simulink model "non_linear_final"  (R2021b) (continued)
== system non_linear_final/Directional Control Valve == (continued)
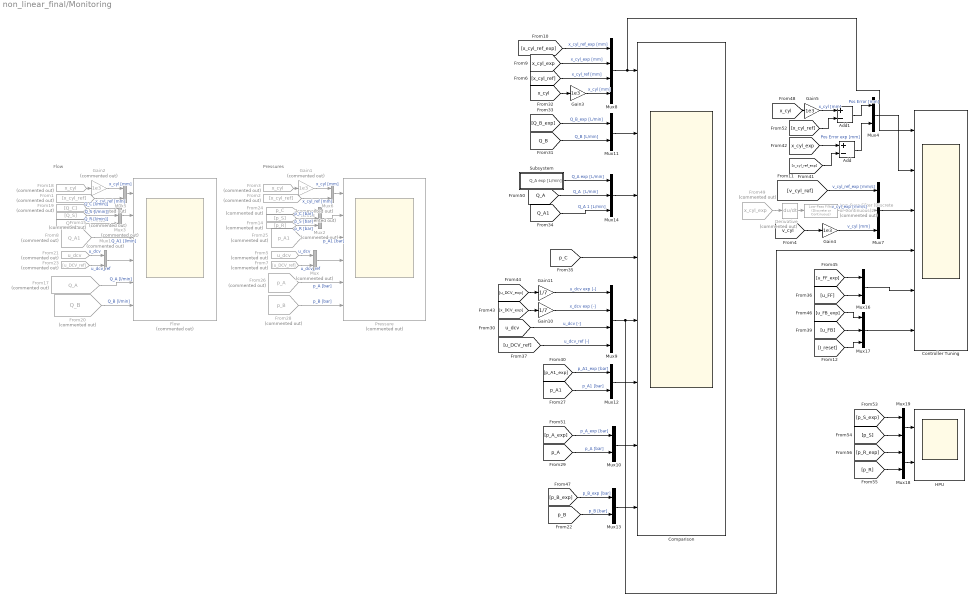
== system non_linear_final/Monitoring ==
  note: Pressures
  note: Flow
  Add : Sum  Inputs=+-
  Add1 : Sum  Inputs=+-
  Comparison : Scope  NumInputPorts=8
  Controller Tuning : Scope  NumInputPorts=6
  Derivative : Derivative  Commented=on
  Flow : Scope  Commented=on  NumInputPorts=6
  From1 : From  Commented=on  GotoTag=x_cyl_ref  TagVisibility=global
  From13 : From  Commented=on  GotoTag=p_S  TagVisibility=global
  From14 : From  Commented=on  GotoTag=p_R  TagVisibility=global
  From15 : From  Commented=on  GotoTag=Q_S  TagVisibility=global
  From16 : From  Commented=on  GotoTag=Q_R  TagVisibility=global
  From17 : From  Commented=on  GotoTag=Q_A  TagVisibility=global
  From18 : From  Commented=on  GotoTag=x_cyl  TagVisibility=global
  From19 : From  Commented=on  GotoTag=Q_C  TagVisibility=global
  From2 : From  Commented=on  GotoTag=x_cyl_ref  TagVisibility=global
  From20 : From  Commented=on  GotoTag=Q_B  TagVisibility=global
  From21 : From  Commented=on  GotoTag=u_dcv  TagVisibility=global
  From23 : From  Commented=on  GotoTag=u_DCV_ref  TagVisibility=global
  From24 : From  Commented=on  GotoTag=p_C  TagVisibility=global
  From25 : From  Commented=on  GotoTag=p_A1  TagVisibility=global
  From26 : From  Commented=on  GotoTag=p_A  TagVisibility=global
  From28 : From  Commented=on  GotoTag=p_B  TagVisibility=global
  From3 : From  Commented=on  GotoTag=x_cyl  TagVisibility=global
  From49 : From  Commented=on  GotoTag=x_cyl_exp  TagVisibility=global
  From5 : From  Commented=on  GotoTag=u_dcv  TagVisibility=global
  From7 : From  Commented=on  GotoTag=u_DCV_ref  TagVisibility=global
  From8 : From  Commented=on  GotoTag=Q_A1  TagVisibility=global
  Gain1 : Gain  Commented=on  Gain=1e3
  Gain10 : Gain  Gain=1/7
  Gain11 : Gain  Gain=1/7
  Gain2 : Gain  Commented=on  Gain=1e3
  Gain3 : Gain  Gain=1e3
  Gain4 : Gain  Gain=1e3
  Gain5 : Gain  Gain=1e3
  HPU : Scope  NumInputPorts=2
  Low-Pass Filter (Discrete or Continuous)2 : eeGeneralControl/Low-Pass Filter (Discrete or Continuous)  Commented=on  K=1  T=0.1  B=150  A=-150  init_option=Inherited from block input  x0=0  Ts=0
  Mux{,1,5..6} (4 alike) : Mux  Commented=on  Inputs=2
  Mux{4,10..13,16,18..19} (8 alike) : Mux  Inputs=2
  Mux14 : Mux  Inputs=3
  Mux17 : Mux  Inputs=3
  Mux2 : Mux  Commented=on  Inputs=3
  Mux3 : Mux  Commented=on  Inputs=3
  Mux7 : Mux  Inputs=3
  Mux8 : Mux
  Mux9 : Mux
  Pressure : Scope  Commented=on  NumInputPorts=6
  Subsystem : Subsystem
  wires:
  From28/1 -> Pressure/6  "p_B [bar]"
  From8/1 -> Flow/3  "Q_A1 [l/min]"
  From21/1 -> Mux1/1  "u_dcv"
  From17/1 -> Flow/5  "Q_A [l/min]"
  From26/1 -> Pressure/5  "p_A [bar]"
  From23/1 -> Mux1/2  "u_dcv_ref"
  From20/1 -> Flow/6  "Q_B [l/min]"
  Mux/1 -> Pressure/4
  From2/1 -> Mux6/2  "x_cyl_ref [mm]"
  From16/1 -> Mux3/3  "Q_R [l/min]]"
  From3/1 -> Gain1/1
  From24/1 -> Mux2/1  "p_C [bar]"
  From7/1 -> Mux/2  "u_dcv_ref"
  Mux1/1 -> Flow/4
  From25/1 -> Pressure/3  "p_A1 [bar]"
  Mux3/1 -> Flow/2
  Mux2/1 -> Pressure/2
  From5/1 -> Mux/1  "u_dcv"
  Mux5/1 -> Flow/1
  From14/1 -> Mux2/3  "p_R [bar]"
  From1/1 -> Mux5/2  "x_cyl_ref [mm]"
  From15/1 -> Mux3/2  "Q_S [l/min]]"
  From18/1 -> Gain2/1
  Gain2/1 -> Mux5/1  "x_cyl [mm]"
  From19/1 -> Mux3/1  "Q_C [l/min]]"
  From13/1 -> Mux2/2  "p_S [bar]"
  Gain1/1 -> Mux6/1  "x_cyl [mm]"
  Mux6/1 -> Pressure/1
  Mux8/1 -> Controller Tuning/1, Comparison/1
  [x_cyl_ref] -> Mux8/3  "x_cyl_ref [mm]"
  [x_cyl] -> Gain3/1
  Gain3/1 -> Mux8/4  "x_cyl [mm]"
  [x_cyl_ref_exp] -> Mux8/1  "x_cyl_ref_exp [mm]"
  [x_cyl_exp] -> Mux8/2  "x_cyl_exp [mm]"
  [Q_B] -> Mux11/2  "Q_B [L/min]"
  [Q_B_exp] -> Mux11/1  "Q_B_exp [L/min]"
  Mux11/1 -> Comparison/2
  [p_R] -> Mux18/2
  [p_R_exp] -> Mux18/1
  Mux18/1 -> HPU/2
  [p_S_exp] -> Mux19/1
  [p_S] -> Mux19/2
  Mux19/1 -> HPU/1
  [p_C] -> Comparison/4
  Mux17/1 -> Controller Tuning/6
  [u_FB] -> Mux17/2
  [u_FF] -> Mux16/2
  [u_FF_exp] -> Mux16/1
  [u_FB_exp] -> Mux17/1
  Mux16/1 -> Controller Tuning/5
  [x_cyl_exp] -> Add/1
  [x_cyl_ref] -> Add1/2
  [x_cyl_ref_exp] -> Add/2
  Gain5/1 -> Add1/1  "x_cyl [mm]"
  [x_cyl] -> Gain5/1
  Add/1 -> Mux4/2  "Pos Error exp [mm]"
  Add1/1 -> Mux4/1  "Pos Error [mm]"
  Mux4/1 -> Controller Tuning/2
  [I_reset] -> Mux17/3
  [x_DCV_exp] -> Gain10/1
  Gain10/1 -> Mux9/2  "x_dcv exp [-]"
  [u_DCV_ref] -> Mux9/4  "u_dcv_ref [-]"
  [u_dcv] -> Mux9/3  "u_dcv [-]"
  Gain11/1 -> Mux9/1  "u_dcv exp [-]"
  [p_A1_exp] -> Mux12/1  "p_A1_exp [bar]"
  [p_A1] -> Mux12/2  "p_A1 [bar]"
  Mux12/1 -> Comparison/6
  Mux9/1 -> Controller Tuning/4, Comparison/5
  Low-Pass Filter (Discrete or Continuous)2/1 -> Mux7/2  "v_cyl_exp [mm/s]"
  Mux7/1 -> Controller Tuning/3
  Derivative/1 -> Low-Pass Filter (Discrete or Continuous)2/1
  [v_cyl_ref] -> Mux7/1  "v_cyl_ref_exp [mm/s]"
  [v_cyl] -> Gain4/1
  From49/1 -> Derivative/1
  Gain4/1 -> Mux7/3  "v_cyl [mm]"
  [Q_A1] -> Mux14/3  "Q_A 1 [L/min]"
  Subsystem/1(Q_A exp [L/min]) -> Mux14/1  "Q_A exp [L/min]"
  [Q_A] -> Mux14/2  "Q_A  [L/min]"
  Mux14/1 -> Comparison/3
  [u_DCV_exp] -> Gain11/1
  [p_B] -> Mux13/2  "p_B [bar]"
  [p_B_exp] -> Mux13/1  "p_B_exp [bar]"
  [p_A] -> Mux10/2  "p_A [bar]"
  [p_A_exp] -> Mux10/1  "p_A_exp [bar]"
  Mux13/1 -> Comparison/8
  Mux10/1 -> Comparison/7

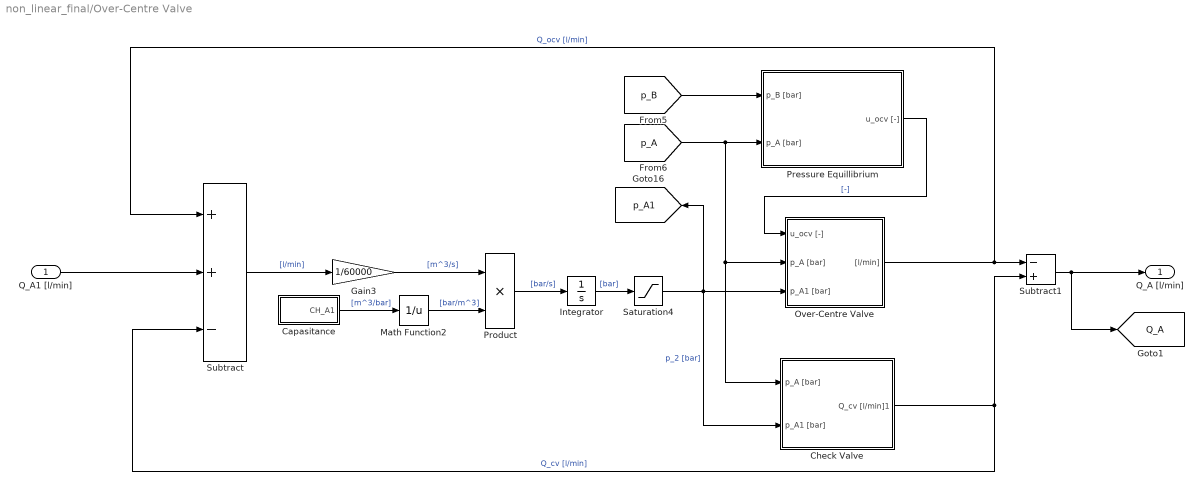
== system non_linear_final/Over-Centre Valve ==
  Q_A1 [l/min] : Inport
  Capasitance : Subsystem
  Check Valve : Subsystem
  Gain3 : Gain  Gain=1/60000
  Integrator : Integrator  InitialCondition=p0_A1
  Math Function2 : Math  Operator=reciprocal  SignedPower=on  SaturateOnIntegerOverflow=on
  Over-Centre Valve : Subsystem
  Pressure Equillibrium : Subsystem
  Product : Product
  Saturation4 : Saturate  UpperLimit=200  LowerLimit=p_R  ZeroCross=off
  Subtract : Sum  Inputs=++-
  Subtract1 : Sum  Inputs=-+
  Q_A [l/min] : Outport  PortDimensions=1  VectorParamsAs1DForOutWhenUnconnected=off
  tag scope: Q_A=global, p_A1=global
  wires:
  [p_B] -> Pressure Equillibrium/1(p_B [bar])
  Pressure Equillibrium/1(u_ocv [-]) -> Over-Centre Valve/1(u_ocv [-])  "[-]"
  Check Valve/1(Q_cv [l/min]1) -> Subtract/3, Subtract1/2  "Q_cv [l/min]"
  Over-Centre Valve/1("[l/min]") -> Subtract1/1, Subtract/1  "Q_ocv [l/min]"
  Saturation4/1 -> Check Valve/2(p_A1 [bar]), Over-Centre Valve/3(p_A1 [bar]), [p_A1]  "p_2 [bar]"
  Subtract/1 -> Gain3/1  "[l/min]"
  Q_A1 [l/min]/1 -> Subtract/2
  Product/1 -> Integrator/1  "[bar/s]"
  Integrator/1 -> Saturation4/1  "[bar]"
  Gain3/1 -> Product/1  "[m^3/s]"
  Subtract1/1 -> [Q_A], Q_A [l/min]/1
  [p_A] -> Pressure Equillibrium/2(p_A [bar]), Over-Centre Valve/2(p_A [bar]), Check Valve/1(p_A [bar])
  Capasitance/1("CH_A1 ") -> Math Function2/1  "[m^3/bar]"
  Math Function2/1 -> Product/2  "[bar/m^3]"

== system non_linear_final/Pressure Compensator1 ==
  p_S [bar] : Inport
  Compensator From Morten Bak Paper : MATLAB Function  TreatAsAtomicUnit=on
  "Compensator setting [bar] " : Constant  Value=p_pc_set
  Transfer Fcn1 : TransferFcn  Denominator=[tau_pc 1]
  p_C [bar] : Outport  VectorParamsAs1DForOutWhenUnconnected=off
  tag scope: p_C=global
  wires:
  [p_LS] -> Compensator From Morten Bak Paper/3(p_LS)
  "Compensator setting [bar] "/1 -> Compensator From Morten Bak Paper/1(p0)
  p_S [bar]/1 -> Compensator From Morten Bak Paper/2(p_S)
  Compensator From Morten Bak Paper/1(p_C) -> Transfer Fcn1/1  "p_C [bar]"
  Transfer Fcn1/1 -> [p_C], p_C [bar]/1  "u_ocv_ref [-]"

== system non_linear_final/Rigid Mechanical System - Green Crane Arm ==
  note: Green Crane (Lagrangian mechanics)
  Force_Input : Inport
  Divide : Product  Inputs=*/
  Lasse Schmidt : MATLAB Function  TreatAsAtomicUnit=on
  Math Function : Math  Operator=square  SignedPower=on  SaturateOnIntegerOverflow=on
  Position : Integrator  InitialCondition=x_cyl_0
  Product : Product
  Scope : Scope
  Sum2 : Sum  Inputs=+--
  Velocity : Integrator  InitialCondition=v_cyl_0
  tag scope: v_cyl=global, x_cyl=global
  wires:
  Velocity/1 -> [v_cyl], Position/1, Math Function/1  "[m/s]"
  Lasse Schmidt/3(Meq) -> Divide/2  "[kg]"
  Sum2/1 -> Divide/1  "[N]"
  Force_Input/1 -> Sum2/1
  Divide/1 -> Velocity/1  "[m/s^2]"
  Lasse Schmidt/1(Geq) -> Sum2/2  "[N]"
  Position/1 -> [x_cyl], Lasse Schmidt/1(x)  "[m]"
  Lasse Schmidt/2(Ceq) -> Product/1
  Math Function/1 -> Product/2
  Product/1 -> Sum2/3, Scope/1  "[N]"

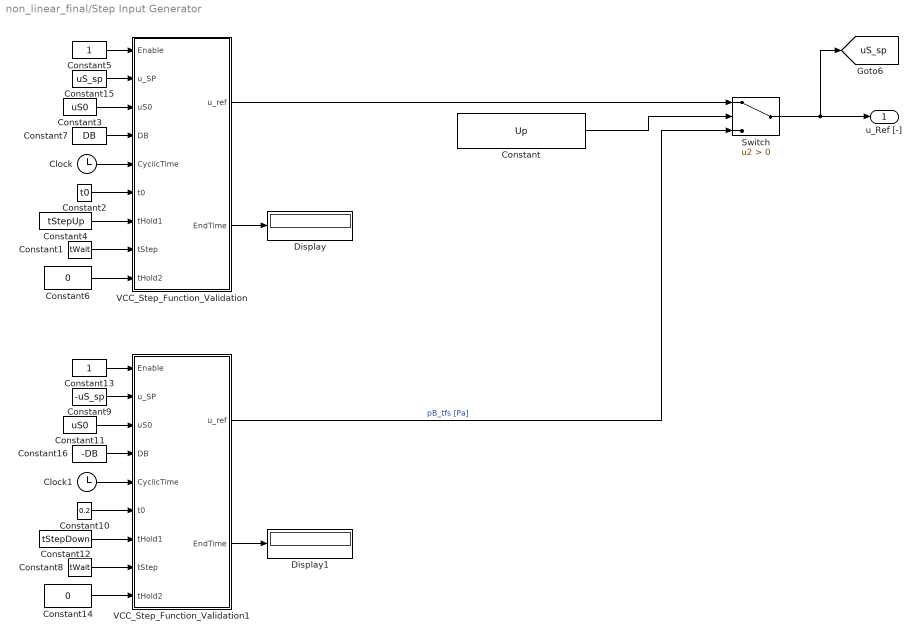
== system non_linear_final/Step Input Generator ==
  Clock : Clock
  Clock1 : Clock
  Constant : Constant  Value=Up
  Constant1 : Constant  Value=tWait
  Constant10 : Constant  Value=0.2
  Constant11 : Constant  Value=uS0
  Constant12 : Constant  Value=tStepDown
  Constant13 : Constant  OutDataTypeStr=boolean
  Constant14 : Constant  Value=0
  Constant15 : Constant  Value=uS_sp
  Constant16 : Constant  Value=-DB
  Constant2 : Constant  Value=t0
  Constant3 : Constant  Value=uS0
  Constant4 : Constant  Value=tStepUp
  Constant5 : Constant  OutDataTypeStr=boolean
  Constant6 : Constant  Value=0
  Constant7 : Constant  Value=DB
  Constant8 : Constant  Value=tWait
  Constant9 : Constant  Value=-uS_sp
  Display : Display
  Display1 : Display
  Switch : Switch  Criteria=u2 > Threshold
  VCC_Step_Function_Validation : Subsystem  TreatAsAtomicUnit=on
  VCC_Step_Function_Validation1 : Subsystem  TreatAsAtomicUnit=on
  u_Ref [-] : Outport  VectorParamsAs1DForOutWhenUnconnected=off
  tag scope: uS_sp=global
  wires:
  VCC_Step_Function_Validation/2(EndTime) -> Display/1
  Constant4/1 -> VCC_Step_Function_Validation/7(tHold1)
  Constant1/1 -> VCC_Step_Function_Validation/8(tStep)
  Clock/1 -> VCC_Step_Function_Validation/5(CyclicTime)
  Constant2/1 -> VCC_Step_Function_Validation/6(t0)
  Constant15/1 -> VCC_Step_Function_Validation/2(u_SP)
  VCC_Step_Function_Validation/1(u_ref) -> Switch/1
  Constant3/1 -> VCC_Step_Function_Validation/3(uS0)
  Constant6/1 -> VCC_Step_Function_Validation/9(tHold2)
  Constant5/1 -> VCC_Step_Function_Validation/1(Enable)
  Constant7/1 -> VCC_Step_Function_Validation/4(DB)
  Switch/1 -> [uS_sp], u_Ref [-]/1
  Constant/1 -> Switch/2
  VCC_Step_Function_Validation1/1(u_ref) -> Switch/3  "pB_tfs [Pa]"
  Constant16/1 -> VCC_Step_Function_Validation1/4(DB)
  Constant13/1 -> VCC_Step_Function_Validation1/1(Enable)
  Constant11/1 -> VCC_Step_Function_Validation1/3(uS0)
  Constant12/1 -> VCC_Step_Function_Validation1/7(tHold1)
  Clock1/1 -> VCC_Step_Function_Validation1/5(CyclicTime)
  VCC_Step_Function_Validation1/2(EndTime) -> Display1/1
  Constant9/1 -> VCC_Step_Function_Validation1/2(u_SP)
  Constant14/1 -> VCC_Step_Function_Validation1/9(tHold2)
  Constant10/1 -> VCC_Step_Function_Validation1/6(t0)
  Constant8/1 -> VCC_Step_Function_Validation1/8(tStep)

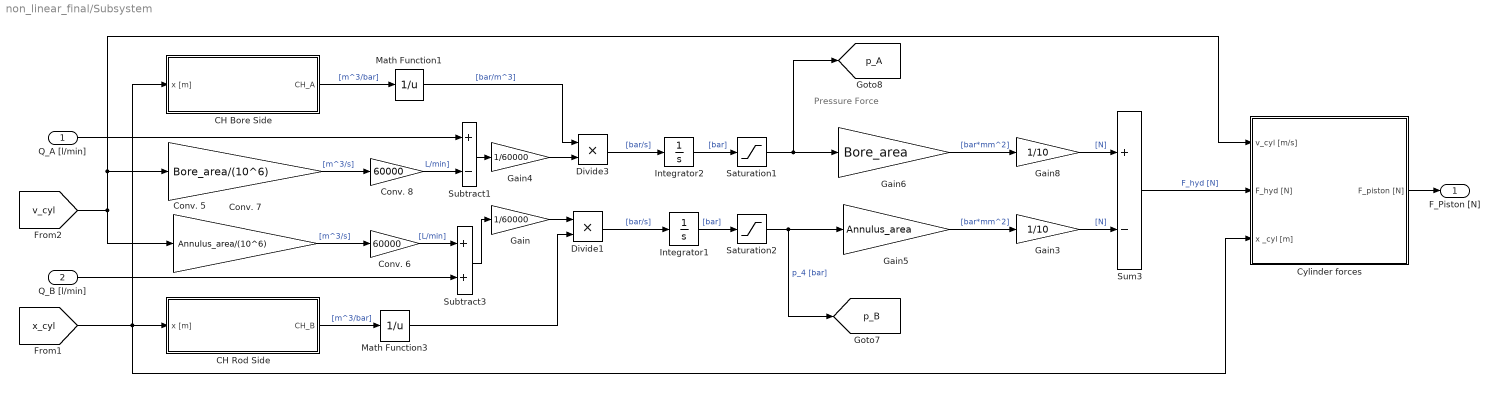
== system non_linear_final/Subsystem ==
  note: Pressure Force
  Q_A [l/min] : Inport
  Q_B [l/min] : Inport  Port=2
  CH Bore Side : Subsystem
  CH Rod Side : Subsystem
  Conv. 5 : Gain  Gain=Annulus_area/(10^6)
  Conv. 6 : Gain  Gain=60000
  Conv. 7 : Gain  Gain=Bore_area/(10^6)
  Conv. 8 : Gain  Gain=60000
  Cylinder forces : Subsystem
  Divide1 : Product  Inputs=**
  Divide3 : Product  Inputs=**
  Gain : Gain  Gain=1/60000
  Gain3 : Gain  Gain=1/10
  Gain4 : Gain  Gain=1/60000
  Gain5 : Gain  Gain=Annulus_area
  Gain6 : Gain  Gain=Bore_area
  Gain8 : Gain  Gain=1/10
  Integrator1 : Integrator  InitialCondition=p0_B  LowerSaturationLimit=0
  Integrator2 : Integrator  InitialCondition=p0_A  LowerSaturationLimit=0
  Math Function1 : Math  Operator=reciprocal  SignedPower=on  SaturateOnIntegerOverflow=on
  Math Function3 : Math  Operator=reciprocal  SignedPower=on  SaturateOnIntegerOverflow=on
  Saturation1 : Saturate  UpperLimit=200  LowerLimit=p_R  ZeroCross=off
  Saturation2 : Saturate  UpperLimit=200  LowerLimit=p_R  ZeroCross=off
  Subtract1 : Sum  Inputs=+-
  Subtract3 : Sum
  Sum3 : Sum  Inputs=+-
  F_Piston [N] : Outport  VectorParamsAs1DForOutWhenUnconnected=off
  tag scope: p_A=global, p_B=global
  wires:
  Sum3/1 -> Cylinder forces/2(F_hyd [N])  "F_hyd [N]"
  Gain8/1 -> Sum3/1  "[N]"
  Gain3/1 -> Sum3/2  "[N]"
  Saturation1/1 -> [p_A], Gain6/1
  Gain5/1 -> Gain3/1  "[bar*mm^2]"
  Gain6/1 -> Gain8/1  "[bar*mm^2]"
  Cylinder forces/1(F_piston [N]) -> F_Piston [N]/1
  [v_cyl] -> Conv. 5/1, Cylinder forces/1(v_cyl [m/s]), Conv. 7/1
  [x_cyl] -> CH Rod Side/1(x [m]), CH Bore Side/1(x [m]), Cylinder forces/3(x _cyl [m])
  Integrator1/1 -> Saturation2/1  "[bar]"
  Q_B [l/min]/1 -> Subtract3/2
  Divide1/1 -> Integrator1/1  "[bar/s]"
  Saturation2/1 -> [p_B], Gain5/1  "p_4 [bar]"
  Gain/1 -> Divide1/1
  Integrator2/1 -> Saturation1/1  "[bar]"
  Gain4/1 -> Divide3/2
  Divide3/1 -> Integrator2/1  "[bar/s]"
  Math Function1/1 -> Divide3/1  "[bar/m^3]"
  CH Bore Side/1(CH_A) -> Math Function1/1  "[m^3/bar]"
  Math Function3/1 -> Divide1/2
  CH Rod Side/1(CH_B) -> Math Function3/1  "[m^3/bar]"
  Subtract3/1 -> Gain/1
  Conv. 6/1 -> Subtract3/1  "[L/min]"
  Conv. 5/1 -> Conv. 6/1  "[m^3/s]"
  Subtract1/1 -> Gain4/1
  Q_A [l/min]/1 -> Subtract1/1
  Conv. 7/1 -> Conv. 8/1  "[m^3/s]"
  Conv. 8/1 -> Subtract1/2  "L/min]"

== system non_linear_final/Subsystem1 ==
  Clock : Clock
  Constant1 : Constant  Value=tWait
  Constant15 : Constant  Value=uS_sp
  Constant2 : Constant  Value=t0
  Constant3 : Constant  Value=uS0
  Constant4 : Constant  Value=tStepUp
  Constant5 : Constant  OutDataTypeStr=boolean
  Constant6 : Constant  Value=tStepDown
  Constant7 : Constant  Value=DB
  Display : Display
  VCC_Step_Function_Validation : Subsystem  TreatAsAtomicUnit=on
  u_Ref [-] : Outport  VectorParamsAs1DForOutWhenUnconnected=off
  tag scope: uS_sp=global
  wires:
  VCC_Step_Function_Validation/2(EndTime) -> Display/1
  Constant4/1 -> VCC_Step_Function_Validation/7(tHold1)
  Constant1/1 -> VCC_Step_Function_Validation/8(tStep)
  Clock/1 -> VCC_Step_Function_Validation/5(CyclicTime)
  Constant2/1 -> VCC_Step_Function_Validation/6(t0)
  Constant15/1 -> VCC_Step_Function_Validation/2(u_SP)
  VCC_Step_Function_Validation/1(u_ref) -> u_Ref [-]/1, [uS_sp]  "pB_tfs [Pa]"
  Constant3/1 -> VCC_Step_Function_Validation/3(uS0)
  Constant6/1 -> VCC_Step_Function_Validation/9(tHold2)
  Constant5/1 -> VCC_Step_Function_Validation/1(Enable)
  Constant7/1 -> VCC_Step_Function_Validation/4(DB)

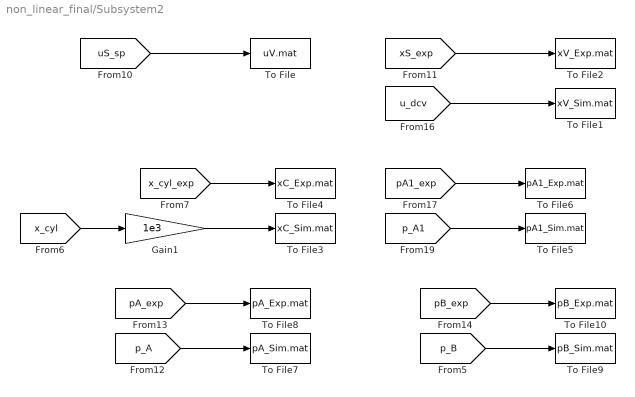
== system non_linear_final/Subsystem2 ==
  Gain1 : Gain  Gain=1e3
  To File : ToFile  Filename=uV.mat  MatrixName=uV  SaveFormat=Timeseries
  To File1 : ToFile  Filename=xV_Sim.mat  MatrixName=xV_Sim  SaveFormat=Timeseries
  To File10 : ToFile  Filename=pB_Exp.mat  MatrixName=pB_Exp  SaveFormat=Timeseries
  To File2 : ToFile  Filename=xV_Exp.mat  MatrixName=xV_Exp  SaveFormat=Timeseries
  To File3 : ToFile  Filename=xC_Sim.mat  MatrixName=xC_Sim  SaveFormat=Timeseries
  To File4 : ToFile  Filename=xC_Exp.mat  MatrixName=xC_Exp  SaveFormat=Timeseries
  To File5 : ToFile  Filename=pA1_Sim.mat  MatrixName=pA1_Sim  SaveFormat=Timeseries
  To File6 : ToFile  Filename=pA1_Exp.mat  MatrixName=pA1_Exp  SaveFormat=Timeseries
  To File7 : ToFile  Filename=pA_Sim.mat  MatrixName=pA_Sim  SaveFormat=Timeseries
  To File8 : ToFile  Filename=pA_Exp.mat  MatrixName=pA_Exp  SaveFormat=Timeseries
  To File9 : ToFile  Filename=pB_Sim.mat  MatrixName=pB_Sim  SaveFormat=Timeseries
  wires:
  [x_cyl] -> Gain1/1
  [uS_sp] -> To File/1
  [xS_exp] -> To File2/1
  [u_dcv] -> To File1/1
  Gain1/1 -> To File3/1
  [x_cyl_exp] -> To File4/1
  [pA1_exp] -> To File6/1
  [p_A1] -> To File5/1
  [pA_exp] -> To File8/1
  [p_A] -> To File7/1
  [p_B] -> To File9/1
  [pB_exp] -> To File10/1

== system non_linear_final/Comparison Exp1/Down ==
  Demux : Demux  Tag=STV Demux  Outputs=9
      settings: DisplayOption=none
  FromWs : FromWorkspace  Tag=STV FromWs  VariableName=tuvar
  Imported_Signal 1 : Outport  Tag=STV Outport  VectorParamsAs1DForOutWhenUnconnected=off
  Imported_Signal 2 : Outport  Tag=STV Outport  Port=2  VectorParamsAs1DForOutWhenUnconnected=off
  Imported_Signal 3 : Outport  Tag=STV Outport  Port=3  VectorParamsAs1DForOutWhenUnconnected=off
  Imported_Signal 4 : Outport  Tag=STV Outport  Port=4  VectorParamsAs1DForOutWhenUnconnected=off
  Imported_Signal 5 : Outport  Tag=STV Outport  Port=5  VectorParamsAs1DForOutWhenUnconnected=off
  Imported_Signal 6 : Outport  Tag=STV Outport  Port=6  VectorParamsAs1DForOutWhenUnconnected=off
  Imported_Signal 7 : Outport  Tag=STV Outport  Port=7  VectorParamsAs1DForOutWhenUnconnected=off
  Imported_Signal 8 : Outport  Tag=STV Outport  Port=8  VectorParamsAs1DForOutWhenUnconnected=off
  Imported_Signal 9 : Outport  Tag=STV Outport  Port=9  VectorParamsAs1DForOutWhenUnconnected=off
  wires:
  Demux/1 -> Imported_Signal 1/1
  FromWs/1 -> Demux/1
  Demux/2 -> Imported_Signal 2/1
  Demux/3 -> Imported_Signal 3/1
  Demux/4 -> Imported_Signal 4/1
  Demux/5 -> Imported_Signal 5/1
  Demux/6 -> Imported_Signal 6/1
  Demux/7 -> Imported_Signal 7/1
  Demux/8 -> Imported_Signal 8/1
  Demux/9 -> Imported_Signal 9/1

== system non_linear_final/Comparison Exp1/Orginal DOwn ==
  Demux : Demux  Tag=STV Demux  Outputs=9
      settings: DisplayOption=none
  FromWs : FromWorkspace  Tag=STV FromWs  VariableName=tuvar
  Imported_Signal 1 : Outport  Tag=STV Outport  VectorParamsAs1DForOutWhenUnconnected=off
  Imported_Signal 2 : Outport  Tag=STV Outport  Port=2  VectorParamsAs1DForOutWhenUnconnected=off
  Imported_Signal 3 : Outport  Tag=STV Outport  Port=3  VectorParamsAs1DForOutWhenUnconnected=off
  Imported_Signal 4 : Outport  Tag=STV Outport  Port=4  VectorParamsAs1DForOutWhenUnconnected=off
  Imported_Signal 5 : Outport  Tag=STV Outport  Port=5  VectorParamsAs1DForOutWhenUnconnected=off
  Imported_Signal 6 : Outport  Tag=STV Outport  Port=6  VectorParamsAs1DForOutWhenUnconnected=off
  Imported_Signal 7 : Outport  Tag=STV Outport  Port=7  VectorParamsAs1DForOutWhenUnconnected=off
  Imported_Signal 8 : Outport  Tag=STV Outport  Port=8  VectorParamsAs1DForOutWhenUnconnected=off
  Imported_Signal 9 : Outport  Tag=STV Outport  Port=9  VectorParamsAs1DForOutWhenUnconnected=off
  wires:
  Demux/1 -> Imported_Signal 1/1
  FromWs/1 -> Demux/1
  Demux/2 -> Imported_Signal 2/1
  Demux/3 -> Imported_Signal 3/1
  Demux/4 -> Imported_Signal 4/1
  Demux/5 -> Imported_Signal 5/1
  Demux/6 -> Imported_Signal 6/1
  Demux/7 -> Imported_Signal 7/1
  Demux/8 -> Imported_Signal 8/1
  Demux/9 -> Imported_Signal 9/1

== system non_linear_final/Comparison Exp1/Orginal DOwn1 ==
  Demux : Demux  Tag=STV Demux  Outputs=9
      settings: DisplayOption=none
  FromWs : FromWorkspace  Tag=STV FromWs  VariableName=tuvar
  Imported_Signal 1 : Outport  Tag=STV Outport  VectorParamsAs1DForOutWhenUnconnected=off
  Imported_Signal 2 : Outport  Tag=STV Outport  Port=2  VectorParamsAs1DForOutWhenUnconnected=off
  Imported_Signal 3 : Outport  Tag=STV Outport  Port=3  VectorParamsAs1DForOutWhenUnconnected=off
  Imported_Signal 4 : Outport  Tag=STV Outport  Port=4  VectorParamsAs1DForOutWhenUnconnected=off
  Imported_Signal 5 : Outport  Tag=STV Outport  Port=5  VectorParamsAs1DForOutWhenUnconnected=off
  Imported_Signal 6 : Outport  Tag=STV Outport  Port=6  VectorParamsAs1DForOutWhenUnconnected=off
  Imported_Signal 7 : Outport  Tag=STV Outport  Port=7  VectorParamsAs1DForOutWhenUnconnected=off
  Imported_Signal 8 : Outport  Tag=STV Outport  Port=8  VectorParamsAs1DForOutWhenUnconnected=off
  Imported_Signal 9 : Outport  Tag=STV Outport  Port=9  VectorParamsAs1DForOutWhenUnconnected=off
  wires:
  Demux/1 -> Imported_Signal 1/1
  FromWs/1 -> Demux/1
  Demux/2 -> Imported_Signal 2/1
  Demux/3 -> Imported_Signal 3/1
  Demux/4 -> Imported_Signal 4/1
  Demux/5 -> Imported_Signal 5/1
  Demux/6 -> Imported_Signal 6/1
  Demux/7 -> Imported_Signal 7/1
  Demux/8 -> Imported_Signal 8/1
  Demux/9 -> Imported_Signal 9/1

== system non_linear_final/Comparison Exp2/Large valve opening = 0.5 ==
  Demux : Demux  Tag=STV Demux  Outputs=9
      settings: DisplayOption=none
  FromWs : FromWorkspace  Tag=STV FromWs  VariableName=tuvar
  Imported_Signal 1 : Outport  Tag=STV Outport  VectorParamsAs1DForOutWhenUnconnected=off
  Imported_Signal 2 : Outport  Tag=STV Outport  Port=2  VectorParamsAs1DForOutWhenUnconnected=off
  Imported_Signal 3 : Outport  Tag=STV Outport  Port=3  VectorParamsAs1DForOutWhenUnconnected=off
  Imported_Signal 4 : Outport  Tag=STV Outport  Port=4  VectorParamsAs1DForOutWhenUnconnected=off
  Imported_Signal 5 : Outport  Tag=STV Outport  Port=5  VectorParamsAs1DForOutWhenUnconnected=off
  Imported_Signal 6 : Outport  Tag=STV Outport  Port=6  VectorParamsAs1DForOutWhenUnconnected=off
  Imported_Signal 7 : Outport  Tag=STV Outport  Port=7  VectorParamsAs1DForOutWhenUnconnected=off
  Imported_Signal 8 : Outport  Tag=STV Outport  Port=8  VectorParamsAs1DForOutWhenUnconnected=off
  Imported_Signal 9 : Outport  Tag=STV Outport  Port=9  VectorParamsAs1DForOutWhenUnconnected=off
  wires:
  Demux/1 -> Imported_Signal 1/1
  FromWs/1 -> Demux/1
  Demux/2 -> Imported_Signal 2/1
  Demux/3 -> Imported_Signal 3/1
  Demux/4 -> Imported_Signal 4/1
  Demux/5 -> Imported_Signal 5/1
  Demux/6 -> Imported_Signal 6/1
  Demux/7 -> Imported_Signal 7/1
  Demux/8 -> Imported_Signal 8/1
  Demux/9 -> Imported_Signal 9/1

== system non_linear_final/Comparison Exp2/Large valve opening = 0.5 Down Only ==
  Demux : Demux  Tag=STV Demux  Outputs=9
      settings: DisplayOption=none
  FromWs : FromWorkspace  Tag=STV FromWs  VariableName=tuvar
  Imported_Signal 1 : Outport  Tag=STV Outport  VectorParamsAs1DForOutWhenUnconnected=off
  Imported_Signal 2 : Outport  Tag=STV Outport  Port=2  VectorParamsAs1DForOutWhenUnconnected=off
  Imported_Signal 3 : Outport  Tag=STV Outport  Port=3  VectorParamsAs1DForOutWhenUnconnected=off
  Imported_Signal 4 : Outport  Tag=STV Outport  Port=4  VectorParamsAs1DForOutWhenUnconnected=off
  Imported_Signal 5 : Outport  Tag=STV Outport  Port=5  VectorParamsAs1DForOutWhenUnconnected=off
  Imported_Signal 6 : Outport  Tag=STV Outport  Port=6  VectorParamsAs1DForOutWhenUnconnected=off
  Imported_Signal 7 : Outport  Tag=STV Outport  Port=7  VectorParamsAs1DForOutWhenUnconnected=off
  Imported_Signal 8 : Outport  Tag=STV Outport  Port=8  VectorParamsAs1DForOutWhenUnconnected=off
  Imported_Signal 9 : Outport  Tag=STV Outport  Port=9  VectorParamsAs1DForOutWhenUnconnected=off
  wires:
  Demux/1 -> Imported_Signal 1/1
  FromWs/1 -> Demux/1
  Demux/2 -> Imported_Signal 2/1
  Demux/3 -> Imported_Signal 3/1
  Demux/4 -> Imported_Signal 4/1
  Demux/5 -> Imported_Signal 5/1
  Demux/6 -> Imported_Signal 6/1
  Demux/7 -> Imported_Signal 7/1
  Demux/8 -> Imported_Signal 8/1
  Demux/9 -> Imported_Signal 9/1

== system non_linear_final/Comparison Exp2/Orginal DOwn ==
  Demux : Demux  Tag=STV Demux  Outputs=9
      settings: DisplayOption=none
  FromWs : FromWorkspace  Tag=STV FromWs  VariableName=tuvar
  Imported_Signal 1 : Outport  Tag=STV Outport  VectorParamsAs1DForOutWhenUnconnected=off
  Imported_Signal 2 : Outport  Tag=STV Outport  Port=2  VectorParamsAs1DForOutWhenUnconnected=off
  Imported_Signal 3 : Outport  Tag=STV Outport  Port=3  VectorParamsAs1DForOutWhenUnconnected=off
  Imported_Signal 4 : Outport  Tag=STV Outport  Port=4  VectorParamsAs1DForOutWhenUnconnected=off
  Imported_Signal 5 : Outport  Tag=STV Outport  Port=5  VectorParamsAs1DForOutWhenUnconnected=off
  Imported_Signal 6 : Outport  Tag=STV Outport  Port=6  VectorParamsAs1DForOutWhenUnconnected=off
  Imported_Signal 7 : Outport  Tag=STV Outport  Port=7  VectorParamsAs1DForOutWhenUnconnected=off
  Imported_Signal 8 : Outport  Tag=STV Outport  Port=8  VectorParamsAs1DForOutWhenUnconnected=off
  Imported_Signal 9 : Outport  Tag=STV Outport  Port=9  VectorParamsAs1DForOutWhenUnconnected=off
  wires:
  Demux/1 -> Imported_Signal 1/1
  FromWs/1 -> Demux/1
  Demux/2 -> Imported_Signal 2/1
  Demux/3 -> Imported_Signal 3/1
  Demux/4 -> Imported_Signal 4/1
  Demux/5 -> Imported_Signal 5/1
  Demux/6 -> Imported_Signal 6/1
  Demux/7 -> Imported_Signal 7/1
  Demux/8 -> Imported_Signal 8/1
  Demux/9 -> Imported_Signal 9/1

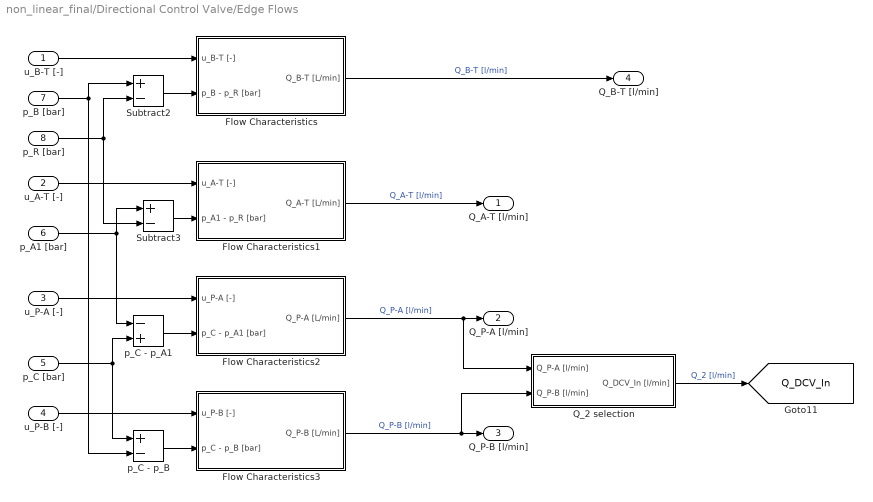
== system non_linear_final/Directional Control Valve/Edge Flows ==
  u_B-T [-] : Inport
  u_A-T [-] : Inport  Port=2
  u_P-A [-] : Inport  Port=3
  u_P-B [-] : Inport  Port=4
  p_C [bar] : Inport  Port=5
  p_A1 [bar] : Inport  Port=6
  p_B [bar] : Inport  Port=7
  p_R [bar] : Inport  Port=8
  Flow Characteristics : Subsystem
  Flow Characteristics1 : Subsystem
  Flow Characteristics2 : Subsystem
  Flow Characteristics3 : Subsystem
  Q_2 selection : Subsystem
  Subtract2 : Sum  Inputs=+-
  Subtract3 : Sum  Inputs=+-
  p_C - p_A1 : Sum  Inputs=-+
  p_C - p_B : Sum  Inputs=+-
  Q_A-T [l/min] : Outport  VectorParamsAs1DForOutWhenUnconnected=off
  Q_P-A [l/min] : Outport  Port=2  VectorParamsAs1DForOutWhenUnconnected=off
  Q_P-B [l/min] : Outport  Port=3  VectorParamsAs1DForOutWhenUnconnected=off
  Q_B-T [l/min] : Outport  Port=4  VectorParamsAs1DForOutWhenUnconnected=off
  tag scope: Q_DCV_In=global
  wires:
  p_C [bar]/1 -> p_C - p_A1/2, p_C - p_B/1
  u_A-T [-]/1 -> Flow Characteristics1/1(u_A-T [-])
  p_A1 [bar]/1 -> Subtract3/1, p_C - p_A1/1
  p_B [bar]/1 -> Subtract2/1, p_C - p_B/2
  p_C - p_B/1 -> Flow Characteristics3/2(p_C - p_B [bar])
  u_P-A [-]/1 -> Flow Characteristics2/1(u_P-A [-])
  p_C - p_A1/1 -> Flow Characteristics2/2(p_C - p_A1 [bar])
  u_P-B [-]/1 -> Flow Characteristics3/1(u_P-B [-])
  p_R [bar]/1 -> Subtract3/2, Subtract2/2
  Flow Characteristics1/1(Q_A-T [L/min]) -> Q_A-T [l/min]/1  "Q_A-T [l/min]"
  Flow Characteristics/1(Q_B-T [L/min]) -> Q_B-T [l/min]/1  "Q_B-T [l/min]"
  Q_2 selection/1(Q_DCV_In [l/min]) -> [Q_DCV_In]  "Q_2 [l/min]"
  Flow Characteristics3/1(Q_P-B [L/min]) -> Q_2 selection/2(Q_P-B [l/min]), Q_P-B [l/min]/1  "Q_P-B [l/min]"
  Flow Characteristics2/1(Q_P-A [L/min]) -> Q_2 selection/1(Q_P-A [l/min]), Q_P-A [l/min]/1  "Q_P-A [l/min]"
  Subtract2/1 -> Flow Characteristics/2(p_B - p_R [bar])
  u_B-T [-]/1 -> Flow Characteristics/1(u_B-T [-])
  Subtract3/1 -> Flow Characteristics1/2(p_A1 - p_R [bar])

== system non_linear_final/Directional Control Valve/Edge Openings ==
  u_dcv [-] : Inport
  Edge A-T : Subsystem
  Edge B-T : Subsystem
  Edge P-A : Subsystem
  Edge P-B : Subsystem
  u_B-T [-] : Outport  VectorParamsAs1DForOutWhenUnconnected=off
  u_A-T [-] : Outport  Port=2  VectorParamsAs1DForOutWhenUnconnected=off
  u_P-A [-] : Outport  Port=3  VectorParamsAs1DForOutWhenUnconnected=off
  u_P-B [-] : Outport  Port=4  VectorParamsAs1DForOutWhenUnconnected=off
  wires:
  Edge A-T/1(u_A-T [-]) -> u_A-T [-]/1  "u_A-T [-]"
  Edge B-T/1(u_B-T [-]) -> u_B-T [-]/1  "u_B-T [-]"
  u_dcv [-]/1 -> Edge P-A/1(u_dcv [-]), Edge P-B/1(u_dcv [-]), Edge A-T/1(u_dcv [-]), Edge B-T/1(u_dcv [-])  "[-]"
  Edge P-A/1(u_P-A [-]) -> u_P-A [-]/1  "u_P-A [-]"
  Edge P-B/1(u_P-B [-]) -> u_P-B [-]/1  "u_P-B [-]"

== system non_linear_final/Directional Control Valve/Load-Sensing Pressure ==
  pB : Inport
  pA1 : Inport  Port=2
  uDCV : Inport  Port=3
  Constant : Constant  Value=0
  Switch : Switch  Criteria=u2 ~= 0
  Switch1 : Switch  Criteria=u2 > Threshold
  pLS : Outport  VectorParamsAs1DForOutWhenUnconnected=off
  wires:
  pA1/1 -> Switch1/3
  Constant/1 -> Switch/3
  Switch1/1 -> Switch/1
  Switch/1 -> pLS/1
  uDCV/1 -> Switch1/2, Switch/2
  pB/1 -> Switch1/1
